simulink model "MX_Gateway"  (Simulink 9.0)
settings: Solver=FixedStepDiscrete  StartTime=0.0  StopTime=100  FixedStep=0.01  RelTol=1e-3
values from neighbouring files (symbols this model uses):
  ACTIVE = Bobcat.Parameter; CreateDefine( ACTIVE                         ,1                          ,'Define' ,'defines.h' )    [defines.m]
  ACTIVE_MODE_ONE = Bobcat.Parameter; CreateDefine( ACTIVE_MODE_ONE                ,19                         ,'Define' ,'defines.h' )    [defines.m]
  AUTHENTICATED = Bobcat.Parameter; CreateDefine( AUTHENTICATED                  ,1                          ,'Define' ,'defines.h' )    [defines.m]
  AUX_EMEA_CONTROLS = Bobcat.Parameter; CreateDefine( AUX_EMEA_CONTROLS  ,1   ,'Define' ,'defines.h' )    [enums.m]
  AUX_FLOW_HIGH = Bobcat.Parameter; CreateDefine( AUX_FLOW_HIGH           ,4  ,'Define' ,'defines.h' )    [enums.m]
  AUX_FLOW_INACTIVE = Bobcat.Parameter; CreateDefine( AUX_FLOW_INACTIVE       ,0  ,'Define' ,'defines.h' )    [enums.m]
  AUX_FLOW_LOW = Bobcat.Parameter; CreateDefine( AUX_FLOW_LOW            ,1  ,'Define' ,'defines.h' )    [enums.m]
  AUX_FLOW_MEDIUM = Bobcat.Parameter; CreateDefine( AUX_FLOW_MEDIUM         ,2  ,'Define' ,'defines.h' )    [enums.m]
  AUX_FLOW_STANDARD = Bobcat.Parameter; CreateDefine( AUX_FLOW_STANDARD       ,3  ,'Define' ,'defines.h' )    [enums.m]
  AUX_NON_FUNCTIONAL = Bobcat.Parameter; CreateDefine( AUX_NON_FUNCTIONAL ,255 ,'Define' ,'defines.h' )    [enums.m]
  BASE_ACTIVE = Bobcat.Parameter; CreateDefine( BASE_ACTIVE                    ,253                        ,'Define' ,'defines.h' )    [defines.m]
  BASE_DETENT = Bobcat.Parameter; CreateDefine( BASE_DETENT                    ,1                          ,'Define' ,'defines.h' )    [defines.m]
  BEEP_NONE = Bobcat.Parameter; CreateDefine( BEEP_NONE                      ,255                        ,'Define' ,'defines.h' )    [defines.m]
  CD_AIR_FILTER_DEBOUNCE_NORMAL = Bobcat.Parameter; CreateAliasCD( CD_AIR_FILTER_DEBOUNCE_NORMAL                        ,uint16(500)                ,'ConstantDataPointer' ,'CONSTANT_DATA' ,'AIR_FILTER_DEBOUNCE_NORMAL')    [cdata.m]    (also in hc12_cdata.m; m4_cdata.m)
  CD_AIR_FILTER_DEBOUNCE_NOT_CONNECTED = Bobcat.Parameter; CreateAliasCD( CD_AIR_FILTER_DEBOUNCE_NOT_CONNECTED                 ,uint16(3000)               ,'ConstantDataPointer' ,'CONSTANT_DATA' ,'AIR_FILTER_DEBOUNCE_NOT_CONNECTED')    [cdata.m]    (also in hc12_cdata.m; m4_cdata.m)
  CD_AIR_FILTER_DEBOUNCE_PLUGGED = Bobcat.Parameter; CreateAliasCD( CD_AIR_FILTER_DEBOUNCE_PLUGGED                       ,uint16(3000)               ,'ConstantDataPointer' ,'CONSTANT_DATA' ,'AIR_FILTER_DEBOUNCE_PLUGGED')    [cdata.m]    (also in hc12_cdata.m; m4_cdata.m)
  CD_AIR_FILTER_FUNCTION = Bobcat.Parameter; CreateAliasCD( CD_AIR_FILTER_FUNCTION                               ,uint8(255)                 ,'ConstantDataPointer' ,'CONSTANT_DATA' ,'AIR_FILTER_FUNCTION')    [cdata.m]    (also in hc12_cdata.m; m4_cdata.m)
  CD_AIR_FILTER_PLUGGED_WEIGHT = Bobcat.Parameter; CreateAliasCD( CD_AIR_FILTER_PLUGGED_WEIGHT                         ,uint8(2)                   ,'ConstantDataPointer' ,'CONSTANT_DATA' ,'AIR_FILTER_PLUGGED_WEIGHT')    [cdata.m]    (also in hc12_cdata.m; m4_cdata.m)
  CD_AIR_FILTER_UNPLUGGED_WEIGHT = Bobcat.Parameter; CreateAliasCD( CD_AIR_FILTER_UNPLUGGED_WEIGHT                       ,uint8(1)                   ,'ConstantDataPointer' ,'CONSTANT_DATA' ,'AIR_FILTER_UNPLUGGED_WEIGHT')    [cdata.m]    (also in hc12_cdata.m; m4_cdata.m)
  CD_AUTOIDLE_ARMBAR_DELAY = Bobcat.Parameter; CreateAliasCD( CD_AUTOIDLE_ARMBAR_DELAY                             ,uint8(2)                   ,'ConstantDataPointer' ,'CONSTANT_DATA' ,'AUTOIDLE_ARMBAR_DELAY')    [cdata.m]    (also in hc12_cdata.m; m4_cdata.m)
  CD_AUTOIDLE_FUNCTION = Bobcat.Parameter; CreateAliasCD( CD_AUTOIDLE_FUNCTION                                 ,uint8(255)                 ,'ConstantDataPointer' ,'CONSTANT_DATA' ,'AUTOIDLE_FUNCTION')    [cdata.m]    (also in hc12_cdata.m; m4_cdata.m)
  CD_AUTOIDLE_IDLE_DELAY = Bobcat.Parameter; CreateAliasCD( CD_AUTOIDLE_IDLE_DELAY                               ,uint8(40)                  ,'ConstantDataPointer' ,'CONSTANT_DATA' ,'AUTOIDLE_IDLE_DELAY')    [cdata.m]    (also in hc12_cdata.m; m4_cdata.m)
  CD_AUTOIDLE_LOW_IDLE_START = Bobcat.Parameter; CreateAliasCD( CD_AUTOIDLE_LOW_IDLE_START                           ,uint8(255)                 ,'ConstantDataPointer' ,'CONSTANT_DATA' ,'AUTOIDLE_LOW_IDLE_START')    [cdata.m]    (also in hc12_cdata.m; m4_cdata.m)
  CD_AUTOIDLE_PRESSURE_OFF = Bobcat.Parameter; CreateAliasCD( CD_AUTOIDLE_PRESSURE_OFF                             ,uint16(300)                ,'ConstantDataPointer' ,'CONSTANT_DATA' ,'AUTOIDLE_PRESSURE_OFF')    [cdata.m]    (also in hc12_cdata.m; m4_cdata.m)
  CD_AUTOIDLE_PRESSURE_ON = Bobcat.Parameter; CreateAliasCD( CD_AUTOIDLE_PRESSURE_ON                              ,uint16(270)                ,'ConstantDataPointer' ,'CONSTANT_DATA' ,'AUTOIDLE_PRESSURE_ON')    [cdata.m]    (also in hc12_cdata.m; m4_cdata.m)
  CD_AUTOSHIFT_FUNCTION = Bobcat.Parameter; CreateAliasCD( CD_AUTOSHIFT_FUNCTION                                ,uint8(255)                 ,'ConstantDataPointer' ,'CONSTANT_DATA' ,'AUTOSHIFT_FUNCTION')    [cdata.m]    (also in hc12_cdata.m; m4_cdata.m)
  CD_AUTOSHIFT_PHIGH1 = Bobcat.Parameter; CreateAliasCD( CD_AUTOSHIFT_PHIGH1                                  ,uint16(205)                ,'ConstantDataPointer' ,'CONSTANT_DATA' ,'AUTOSHIFT_PHIGH1')    [cdata.m]    (also in hc12_cdata.m; m4_cdata.m)
  CD_AUTOSHIFT_PHIGH2 = Bobcat.Parameter; CreateAliasCD( CD_AUTOSHIFT_PHIGH2                                  ,uint16(193)                ,'ConstantDataPointer' ,'CONSTANT_DATA' ,'AUTOSHIFT_PHIGH2')    [cdata.m]    (also in hc12_cdata.m; m4_cdata.m)
  CD_AUTOSHIFT_PHIGH_TEMP1 = Bobcat.Parameter; CreateAliasCD( CD_AUTOSHIFT_PHIGH_TEMP1                             ,int16(70)                  ,'ConstantDataPointer' ,'CONSTANT_DATA' ,'AUTOSHIFT_PHIGH_TEMP1')    [cdata.m]    (also in hc12_cdata.m; m4_cdata.m)
  CD_AUTOSHIFT_PHIGH_TEMP2 = Bobcat.Parameter; CreateAliasCD( CD_AUTOSHIFT_PHIGH_TEMP2                             ,int16(160)                 ,'ConstantDataPointer' ,'CONSTANT_DATA' ,'AUTOSHIFT_PHIGH_TEMP2')    [cdata.m]    (also in hc12_cdata.m; m4_cdata.m)
  CD_AUTOSHIFT_PLOW1 = Bobcat.Parameter; CreateAliasCD( CD_AUTOSHIFT_PLOW1                                   ,uint16(130)                ,'ConstantDataPointer' ,'CONSTANT_DATA' ,'AUTOSHIFT_PLOW1')    [cdata.m]    (also in hc12_cdata.m; m4_cdata.m)
  CD_AUTOSHIFT_PLOW2 = Bobcat.Parameter; CreateAliasCD( CD_AUTOSHIFT_PLOW2                                   ,uint16(100)                ,'ConstantDataPointer' ,'CONSTANT_DATA' ,'AUTOSHIFT_PLOW2')    [cdata.m]    (also in hc12_cdata.m; m4_cdata.m)
  CD_AUTOSHIFT_PLOW_TEMP1 = Bobcat.Parameter; CreateAliasCD( CD_AUTOSHIFT_PLOW_TEMP1                              ,int16(20)                  ,'ConstantDataPointer' ,'CONSTANT_DATA' ,'AUTOSHIFT_PLOW_TEMP1')    [cdata.m]    (also in hc12_cdata.m; m4_cdata.m)
  CD_AUTOSHIFT_PLOW_TEMP2 = Bobcat.Parameter; CreateAliasCD( CD_AUTOSHIFT_PLOW_TEMP2                              ,int16(80)                  ,'ConstantDataPointer' ,'CONSTANT_DATA' ,'AUTOSHIFT_PLOW_TEMP2')    [cdata.m]    (also in hc12_cdata.m; m4_cdata.m)
  CD_AUTOSHIFT_TRAVEL_DELAY = Bobcat.Parameter; CreateAliasCD( CD_AUTOSHIFT_TRAVEL_DELAY                            ,uint8(32)                  ,'ConstantDataPointer' ,'CONSTANT_DATA' ,'AUTOSHIFT_TRAVEL_DELAY')    [cdata.m]    (also in hc12_cdata.m; m4_cdata.m)
  CD_AUX2_CRACKPOINT_CURRENT = Bobcat.Parameter; CreateAliasCD( CD_AUX2_CRACKPOINT_CURRENT                           ,fi(0,0,16,12,'hex','0c28') ,'ConstantDataPointer' ,'CONSTANT_DATA' ,'AUX2_CRACKPOINT_CURRENT')    [cdata.m]    (also in hc12_cdata.m; m4_cdata.m)
  CD_AUX2_FLOW_LOW_FUNCTION = Bobcat.Parameter; CreateAliasCD( CD_AUX2_FLOW_LOW_FUNCTION                            ,uint8(255)                 ,'ConstantDataPointer' ,'CONSTANT_DATA' ,'AUX2_FLOW_LOW_FUNCTION')    [cdata.m]    (also in hc12_cdata.m; m4_cdata.m)
  CD_AUX2_FLOW_MED_FUNCTION = Bobcat.Parameter; CreateAliasCD( CD_AUX2_FLOW_MED_FUNCTION                            ,uint8(255)                 ,'ConstantDataPointer' ,'CONSTANT_DATA' ,'AUX2_FLOW_MED_FUNCTION')    [cdata.m]    (also in hc12_cdata.m; m4_cdata.m)
  CD_AUX2_HI_FLOW_PERCENT = Bobcat.Parameter; CreateAliasCD( CD_AUX2_HI_FLOW_PERCENT                              ,fi(0,0,16,16,'hex','ffff') ,'ConstantDataPointer' ,'CONSTANT_DATA' ,'AUX2_HI_FLOW_PERCENT')    [cdata.m]    (also in hc12_cdata.m; m4_cdata.m)
  CD_AUX2_LOW_FLOW_PERCENT = Bobcat.Parameter; CreateAliasCD( CD_AUX2_LOW_FLOW_PERCENT                             ,fi(0,0,16,16,'hex','7333') ,'ConstantDataPointer' ,'CONSTANT_DATA' ,'AUX2_LOW_FLOW_PERCENT')    [cdata.m]    (also in hc12_cdata.m; m4_cdata.m)
  CD_AUX2_MAX_FLOW_CURRENT = Bobcat.Parameter; CreateAliasCD( CD_AUX2_MAX_FLOW_CURRENT                             ,fi(0,0,16,12,'hex','14cc') ,'ConstantDataPointer' ,'CONSTANT_DATA' ,'AUX2_MAX_FLOW_CURRENT')    [cdata.m]    (also in hc12_cdata.m; m4_cdata.m)
  CD_AUX2_MED_FLOW_PERCENT = Bobcat.Parameter; CreateAliasCD( CD_AUX2_MED_FLOW_PERCENT                             ,fi(0,0,16,16,'hex','9999') ,'ConstantDataPointer' ,'CONSTANT_DATA' ,'AUX2_MED_FLOW_PERCENT')    [cdata.m]    (also in hc12_cdata.m; m4_cdata.m)
  CD_AUX_CRACKPOINT_CURRENT = Bobcat.Parameter; CreateAliasCD( CD_AUX_CRACKPOINT_CURRENT                            ,fi(0,0,16,12,'hex','0000') ,'ConstantDataPointer' ,'CONSTANT_DATA' ,'AUX_CRACKPOINT_CURRENT')    [cdata.m]    (also in hc12_cdata.m; m4_cdata.m)
  CD_AUX_DETENT_FLASH_TIME = Bobcat.Parameter; CreateAliasCD( CD_AUX_DETENT_FLASH_TIME                             ,uint16(72)                 ,'ConstantDataPointer' ,'CONSTANT_DATA' ,'AUX_DETENT_FLASH_TIME')    [cdata.m]    (also in hc12_cdata.m; m4_cdata.m)
  CD_AUX_DETENT_HOLD_TIME = Bobcat.Parameter; CreateAliasCD( CD_AUX_DETENT_HOLD_TIME                              ,uint16(100)                ,'ConstantDataPointer' ,'CONSTANT_DATA' ,'AUX_DETENT_HOLD_TIME')    [cdata.m]    (also in hc12_cdata.m; m4_cdata.m)
  CD_AUX_FULL_ON_AUX1 = Bobcat.Parameter; CreateAliasCD( CD_AUX_FULL_ON_AUX1                                  ,fi(0,0,16,12,'hex','10cd') ,'ConstantDataPointer' ,'CONSTANT_DATA' ,'AUX_FULL_ON_AUX1')    [cdata.m]    (also in hc12_cdata.m; m4_cdata.m)
  CD_AUX_FULL_ON_AUX2 = Bobcat.Parameter; CreateAliasCD( CD_AUX_FULL_ON_AUX2                                  ,fi(0,0,16,12,'hex','139d') ,'ConstantDataPointer' ,'CONSTANT_DATA' ,'AUX_FULL_ON_AUX2')    [cdata.m]    (also in hc12_cdata.m; m4_cdata.m)
  CD_AUX_FULL_ON_AUX3 = Bobcat.Parameter; CreateAliasCD( CD_AUX_FULL_ON_AUX3                                  ,fi(0,0,16,12,'hex','17d7') ,'ConstantDataPointer' ,'CONSTANT_DATA' ,'AUX_FULL_ON_AUX3')    [cdata.m]    (also in hc12_cdata.m; m4_cdata.m)
  CD_AUX_FUNCTION = Bobcat.Parameter; CreateAliasCD( CD_AUX_FUNCTION                                      ,uint8(0)                   ,'ConstantDataPointer' ,'CONSTANT_DATA' ,'AUX_FUNCTION')    [cdata.m]    (also in hc12_cdata.m; m4_cdata.m)
  CD_AUX_RATE_LIMIT_CRACKPOINT = Bobcat.Parameter; CreateAliasCD( CD_AUX_RATE_LIMIT_CRACKPOINT                         ,fi(0,0,16,12,'hex','0800') ,'ConstantDataPointer' ,'CONSTANT_DATA' ,'AUX_RATE_LIMIT_CRACKPOINT')    [cdata.m]    (also in hc12_cdata.m; m4_cdata.m)
  CD_AUX_RATE_LIMIT_CRACKPOINT_RATE = Bobcat.Parameter; CreateAliasCD( CD_AUX_RATE_LIMIT_CRACKPOINT_RATE                    ,fi(0,1,32,12,'int',102400) ,'ConstantDataPointer' ,'CONSTANT_DATA' ,'AUX_RATE_LIMIT_CRACKPOINT_RATE')    [cdata.m]    (also in hc12_cdata.m; m4_cdata.m)
  CD_AUX_RATE_LIMIT_FUNCTION = Bobcat.Parameter; CreateAliasCD( CD_AUX_RATE_LIMIT_FUNCTION                           ,uint8(255)                 ,'ConstantDataPointer' ,'CONSTANT_DATA' ,'AUX_RATE_LIMIT_FUNCTION')    [cdata.m]    (also in hc12_cdata.m; m4_cdata.m)
  CD_AUX_RATE_LIMIT_MAX_ENG_SPD = Bobcat.Parameter; CreateAliasCD( CD_AUX_RATE_LIMIT_MAX_ENG_SPD                        ,uint16(1600)               ,'ConstantDataPointer' ,'CONSTANT_DATA' ,'AUX_RATE_LIMIT_MAX_ENG_SPD')    [cdata.m]    (also in hc12_cdata.m; m4_cdata.m)
  CD_AUX_RATE_LIMIT_MIN_ENG_SPD = Bobcat.Parameter; CreateAliasCD( CD_AUX_RATE_LIMIT_MIN_ENG_SPD                        ,uint16(100)                ,'ConstantDataPointer' ,'CONSTANT_DATA' ,'AUX_RATE_LIMIT_MIN_ENG_SPD')    [cdata.m]    (also in hc12_cdata.m; m4_cdata.m)
  CD_AUX_RATE_LIMIT_NORMAL_RATE = Bobcat.Parameter; CreateAliasCD( CD_AUX_RATE_LIMIT_NORMAL_RATE                        ,fi(0,1,32,12,'int',2048)   ,'ConstantDataPointer' ,'CONSTANT_DATA' ,'AUX_RATE_LIMIT_NORMAL_RATE')    [cdata.m]    (also in hc12_cdata.m; m4_cdata.m)
  CD_AUXPOT_CAL_DEADBAND_HIGH = Bobcat.Parameter; CreateAliasCD( CD_AUXPOT_CAL_DEADBAND_HIGH                          ,uint16(574)                ,'ConstantDataPointer' ,'CONSTANT_DATA' ,'AUXPOT_CAL_DEADBAND_HIGH')    [cdata.m]    (also in hc12_cdata.m; m4_cdata.m)
  CD_AUXPOT_CAL_DEADBAND_LOW = Bobcat.Parameter; CreateAliasCD( CD_AUXPOT_CAL_DEADBAND_LOW                           ,uint16(424)                ,'ConstantDataPointer' ,'CONSTANT_DATA' ,'AUXPOT_CAL_DEADBAND_LOW')    [cdata.m]    (also in hc12_cdata.m; m4_cdata.m)
  CD_AUXPOT_CENTER_RATIO = Bobcat.Parameter; CreateAliasCD( CD_AUXPOT_CENTER_RATIO                               ,uint16(500)                ,'ConstantDataPointer' ,'CONSTANT_DATA' ,'AUXPOT_CENTER_RATIO')    [cdata.m]    (also in hc12_cdata.m; m4_cdata.m)
  CD_AUXPOT_DEADBAND = Bobcat.Parameter; CreateAliasCD( CD_AUXPOT_DEADBAND                                   ,uint8(38)                  ,'ConstantDataPointer' ,'CONSTANT_DATA' ,'AUXPOT_DEADBAND')    [cdata.m]    (also in hc12_cdata.m; m4_cdata.m)
  CD_AUXPOT_FUNCTION = Bobcat.Parameter; CreateAliasCD( CD_AUXPOT_FUNCTION                                   ,uint8(0)                   ,'ConstantDataPointer' ,'CONSTANT_DATA' ,'AUXPOT_FUNCTION')    [cdata.m]    (also in hc12_cdata.m; m4_cdata.m)
  CD_AUXPOT_RATIO_GAIN = Bobcat.Parameter; CreateAliasCD( CD_AUXPOT_RATIO_GAIN                                 ,uint16(1000)               ,'ConstantDataPointer' ,'CONSTANT_DATA' ,'AUXPOT_RATIO_GAIN')    [cdata.m]    (also in hc12_cdata.m; m4_cdata.m)
  CD_CAN_THROTTLE_FUNCTION = Bobcat.Parameter; CreateAliasCD( CD_CAN_THROTTLE_FUNCTION                             ,uint8(255)                 ,'ConstantDataPointer' ,'CONSTANT_DATA' ,'CAN_THROTTLE_FUNCTION')    [cdata.m]    (also in hc12_cdata.m; m4_cdata.m)
  CD_CAN_THROTTLE_REVERSE = Bobcat.Parameter; CreateAliasCD( CD_CAN_THROTTLE_REVERSE                              ,uint8(255)                 ,'ConstantDataPointer' ,'CONSTANT_DATA' ,'CAN_THROTTLE_REVERSE')    [cdata.m]    (also in hc12_cdata.m; m4_cdata.m)
  CD_CONSOLE_DEBOUNCE_ABSENT = Bobcat.Parameter; CreateAliasCD( CD_CONSOLE_DEBOUNCE_ABSENT                           ,uint16(12)                 ,'ConstantDataPointer' ,'CONSTANT_DATA' ,'CONSOLE_DEBOUNCE_ABSENT')    [cdata.m]    (also in hc12_cdata.m; m4_cdata.m)
  CD_CONSOLE_DEBOUNCE_OORH = Bobcat.Parameter; CreateAliasCD( CD_CONSOLE_DEBOUNCE_OORH                             ,uint16(12)                 ,'ConstantDataPointer' ,'CONSTANT_DATA' ,'CONSOLE_DEBOUNCE_OORH')    [cdata.m]    (also in hc12_cdata.m; m4_cdata.m)
  CD_CONSOLE_DEBOUNCE_OORL = Bobcat.Parameter; CreateAliasCD( CD_CONSOLE_DEBOUNCE_OORL                             ,uint16(12)                 ,'ConstantDataPointer' ,'CONSTANT_DATA' ,'CONSOLE_DEBOUNCE_OORL')    [cdata.m]    (also in hc12_cdata.m; m4_cdata.m)
  CD_CONSOLE_DEBOUNCE_PRESENT = Bobcat.Parameter; CreateAliasCD( CD_CONSOLE_DEBOUNCE_PRESENT                          ,uint16(12)                 ,'ConstantDataPointer' ,'CONSTANT_DATA' ,'CONSOLE_DEBOUNCE_PRESENT')    [cdata.m]    (also in hc12_cdata.m; m4_cdata.m)
  CD_CONSOLE_FUNCTION = Bobcat.Parameter; CreateAliasCD( CD_CONSOLE_FUNCTION                                  ,uint8(0)                   ,'ConstantDataPointer' ,'CONSTANT_DATA' ,'CONSOLE_FUNCTION')    [cdata.m]    (also in hc12_cdata.m; m4_cdata.m)
  CD_CONSOLE_OORH = Bobcat.Parameter; CreateAliasCD( CD_CONSOLE_OORH                                      ,uint16(740)                ,'ConstantDataPointer' ,'CONSTANT_DATA' ,'CONSOLE_OORH')    [cdata.m]    (also in hc12_cdata.m; m4_cdata.m)
  CD_CONSOLE_OORL = Bobcat.Parameter; CreateAliasCD( CD_CONSOLE_OORL                                      ,uint16(10)                 ,'ConstantDataPointer' ,'CONSTANT_DATA' ,'CONSOLE_OORL')    [cdata.m]    (also in hc12_cdata.m; m4_cdata.m)
  CD_CONSOLE_PRESENT_HIGH = Bobcat.Parameter; CreateAliasCD( CD_CONSOLE_PRESENT_HIGH                              ,uint16(515)                ,'ConstantDataPointer' ,'CONSTANT_DATA' ,'CONSOLE_PRESENT_HIGH')    [cdata.m]    (also in hc12_cdata.m; m4_cdata.m)
  CD_CONSOLE_PRESENT_LOW = Bobcat.Parameter; CreateAliasCD( CD_CONSOLE_PRESENT_LOW                               ,uint16(375)                ,'ConstantDataPointer' ,'CONSTANT_DATA' ,'CONSOLE_PRESENT_LOW')    [cdata.m]    (also in hc12_cdata.m; m4_cdata.m)
  CD_DELUXE_GEN5_5_FUNCTION = Bobcat.Parameter; CreateAliasCD( CD_DELUXE_GEN5_5_FUNCTION                            ,uint8(255)                 ,'ConstantDataPointer' ,'CONSTANT_DATA' ,'DELUXE_GEN5_5_FUNCTION')    [cdata.m]    (also in hc12_cdata.m; m4_cdata.m)
  CD_DELUXE_GEN5_FUNCTION = Bobcat.Parameter; CreateAliasCD( CD_DELUXE_GEN5_FUNCTION                              ,uint8(255)                 ,'ConstantDataPointer' ,'CONSTANT_DATA' ,'DELUXE_GEN5_FUNCTION')    [cdata.m]    (also in hc12_cdata.m; m4_cdata.m)
  CD_DERATE_ENGINE_SPEED_DEBOUNCE_HIGH = Bobcat.Parameter; CreateAliasCD( CD_DERATE_ENGINE_SPEED_DEBOUNCE_HIGH                 ,uint16(200)                ,'ConstantDataPointer' ,'CONSTANT_DATA' ,'DERATE_ENGINE_SPEED_DEBOUNCE_HIGH')    [cdata.m]    (also in hc12_cdata.m; m4_cdata.m)
  CD_DERATE_ENGINE_SPEED_DEBOUNCE_SHUTDOWN = Bobcat.Parameter; CreateAliasCD( CD_DERATE_ENGINE_SPEED_DEBOUNCE_SHUTDOWN             ,uint16(1000)               ,'ConstantDataPointer' ,'CONSTANT_DATA' ,'DERATE_ENGINE_SPEED_DEBOUNCE_SHUTDOWN')    [cdata.m]    (also in hc12_cdata.m; m4_cdata.m)
  CD_DERATE_ENGINE_SPEED_HIGH_OFFSET = Bobcat.Parameter; CreateAliasCD( CD_DERATE_ENGINE_SPEED_HIGH_OFFSET                   ,uint16(200)                ,'ConstantDataPointer' ,'CONSTANT_DATA' ,'DERATE_ENGINE_SPEED_HIGH_OFFSET')    [cdata.m]    (also in hc12_cdata.m; m4_cdata.m)
  CD_DERATE_METHOD = Bobcat.Parameter; CreateAliasCD( CD_DERATE_METHOD                                     ,uint8(0)                   ,'ConstantDataPointer' ,'CONSTANT_DATA' ,'DERATE_METHOD')    [cdata.m]    (also in hc12_cdata.m; m4_cdata.m)
  CD_DUMP_VALVE_FUNCTION = Bobcat.Parameter; CreateAliasCD( CD_DUMP_VALVE_FUNCTION                               ,uint8(255)                 ,'ConstantDataPointer' ,'CONSTANT_DATA' ,'DUMP_VALVE_FUNCTION')    [cdata.m]    (also in hc12_cdata.m; m4_cdata.m)
  CD_DUMP_VALVE_ON_CURRENT = Bobcat.Parameter; CreateAliasCD( CD_DUMP_VALVE_ON_CURRENT                             ,fi(0,0,16,12,'hex','ffff') ,'ConstantDataPointer' ,'CONSTANT_DATA' ,'DUMP_VALVE_ON_CURRENT')    [cdata.m]    (also in hc12_cdata.m; m4_cdata.m)
  CD_ECO_MODE_THROTTLE_REDUCTION = Bobcat.Parameter; CreateAliasCD( CD_ECO_MODE_THROTTLE_REDUCTION                       ,uint16(850)                ,'ConstantDataPointer' ,'CONSTANT_DATA' ,'ECO_MODE_THROTTLE_REDUCTION')    [cdata.m]    (also in hc12_cdata.m; m4_cdata.m)
  CD_ECT_FILTER_GAIN = Bobcat.Parameter; CreateAliasCD( CD_ECT_FILTER_GAIN                                   ,uint8(6)                   ,'ConstantDataPointer' ,'CONSTANT_DATA' ,'ECT_FILTER_GAIN')    [cdata.m]    (also in hc12_cdata.m; m4_cdata.m)
  CD_ECU_FUNCTION = Bobcat.Parameter; CreateAliasCD( CD_ECU_FUNCTION                                      ,uint8(255)                 ,'ConstantDataPointer' ,'CONSTANT_DATA' ,'ECU_FUNCTION')    [cdata.m]    (also in hc12_cdata.m; m4_cdata.m)
  CD_ECU_HOLD_DEBOUNCE_ERROR_ON = Bobcat.Parameter; CreateAliasCD( CD_ECU_HOLD_DEBOUNCE_ERROR_ON                        ,uint16(20)                 ,'ConstantDataPointer' ,'CONSTANT_DATA' ,'ECU_HOLD_DEBOUNCE_ERROR_ON')    [cdata.m]    (also in hc12_cdata.m; m4_cdata.m)
  CD_EMEA_CONTROLS_HOLD_TIME = Bobcat.Parameter; CreateAliasCD( CD_EMEA_CONTROLS_HOLD_TIME                           ,uint16(30)                 ,'ConstantDataPointer' ,'CONSTANT_DATA' ,'EMEA_CONTROLS_HOLD_TIME')    [cdata.m]    (also in hc12_cdata.m; m4_cdata.m)
  CD_EMEA_CONTROLS_OPTION_A = Bobcat.Parameter; CreateAliasCD( CD_EMEA_CONTROLS_OPTION_A                            ,uint8(255)                 ,'ConstantDataPointer' ,'CONSTANT_DATA' ,'EMEA_CONTROLS_OPTION_A')    [cdata.m]    (also in hc12_cdata.m; m4_cdata.m)
  CD_EMEA_CONTROLS_OPTION_C = Bobcat.Parameter; CreateAliasCD( CD_EMEA_CONTROLS_OPTION_C                            ,uint8(255)                 ,'ConstantDataPointer' ,'CONSTANT_DATA' ,'EMEA_CONTROLS_OPTION_C')    [cdata.m]    (also in hc12_cdata.m; m4_cdata.m)
  CD_EMEA_CONTROLS_REVERSE = Bobcat.Parameter; CreateAliasCD( CD_EMEA_CONTROLS_REVERSE                             ,uint8(255)                 ,'ConstantDataPointer' ,'CONSTANT_DATA' ,'EMEA_CONTROLS_REVERSE')    [cdata.m]    (also in hc12_cdata.m; m4_cdata.m)
  CD_EMEA_ELECTRIC_VERSION = Bobcat.Parameter; CreateAliasCD( CD_EMEA_ELECTRIC_VERSION                             ,uint8(0)                   ,'ConstantDataPointer' ,'CONSTANT_DATA' ,'EMEA_ELECTRIC_VERSION')    [cdata.m]    (also in hc12_cdata.m; m4_cdata.m)
  CD_EMEA_POT_CAL_DEADBAND_HIGH = Bobcat.Parameter; CreateAliasCD( CD_EMEA_POT_CAL_DEADBAND_HIGH                        ,uint16(574)                ,'ConstantDataPointer' ,'CONSTANT_DATA' ,'EMEA_POT_CAL_DEADBAND_HIGH')    [cdata.m]    (also in hc12_cdata.m; m4_cdata.m)
  CD_EMEA_POT_CAL_DEADBAND_LOW = Bobcat.Parameter; CreateAliasCD( CD_EMEA_POT_CAL_DEADBAND_LOW                         ,uint16(424)                ,'ConstantDataPointer' ,'CONSTANT_DATA' ,'EMEA_POT_CAL_DEADBAND_LOW')    [cdata.m]    (also in hc12_cdata.m; m4_cdata.m)
  CD_ENGINE_RPM_FACTOR = Bobcat.Parameter; CreateAliasCD( CD_ENGINE_RPM_FACTOR                                 ,uint8(35)                  ,'ConstantDataPointer' ,'CONSTANT_DATA' ,'ENGINE_RPM_FACTOR')    [cdata.m]    (also in hc12_cdata.m; m4_cdata.m)
  CD_ENGINE_SPEED_FILTER_FAST_VALUE = Bobcat.Parameter; CreateAliasCD( CD_ENGINE_SPEED_FILTER_FAST_VALUE                    ,uint8(1)                   ,'ConstantDataPointer' ,'CONSTANT_DATA' ,'ENGINE_SPEED_FILTER_FAST_VALUE')    [cdata.m]    (also in hc12_cdata.m; m4_cdata.m)
  CD_ENGINE_SPEED_FILTER_SLOW_VALUE = Bobcat.Parameter; CreateAliasCD( CD_ENGINE_SPEED_FILTER_SLOW_VALUE                    ,uint8(4)                   ,'ConstantDataPointer' ,'CONSTANT_DATA' ,'ENGINE_SPEED_FILTER_SLOW_VALUE')    [cdata.m]    (also in hc12_cdata.m; m4_cdata.m)
  CD_ENGINE_SPEED_RUNNING = Bobcat.Parameter; CreateAliasCD( CD_ENGINE_SPEED_RUNNING                              ,uint16(800)                ,'ConstantDataPointer' ,'CONSTANT_DATA' ,'ENGINE_SPEED_RUNNING')    [cdata.m]    (also in hc12_cdata.m; m4_cdata.m)
  CD_ENGINE_START_DELAY_DEBOUNCE_RPM_NO_SIGNAL = Bobcat.Parameter; CreateAliasCD( CD_ENGINE_START_DELAY_DEBOUNCE_RPM_NO_SIGNAL         ,uint16(0)                  ,'ConstantDataPointer' ,'CONSTANT_DATA' ,'ENGINE_START_DELAY_DEBOUNCE_RPM_NO_SIGNAL')    [cdata.m]    (also in hc12_cdata.m; m4_cdata.m)
  CD_ENGINE_START_DELAY_OFF_TIME = Bobcat.Parameter; CreateAliasCD( CD_ENGINE_START_DELAY_OFF_TIME                       ,uint16(0)                  ,'ConstantDataPointer' ,'CONSTANT_DATA' ,'ENGINE_START_DELAY_OFF_TIME')    [cdata.m]    (also in hc12_cdata.m; m4_cdata.m)
  CD_ENGINE_START_DELAY_RPM_NO_SIGNAL = Bobcat.Parameter; CreateAliasCD( CD_ENGINE_START_DELAY_RPM_NO_SIGNAL                  ,uint16(0)                  ,'ConstantDataPointer' ,'CONSTANT_DATA' ,'ENGINE_START_DELAY_RPM_NO_SIGNAL')    [cdata.m]    (also in hc12_cdata.m; m4_cdata.m)
  CD_EOP_DEBOUNCE_EXTREMELY_LOW = Bobcat.Parameter; CreateAliasCD( CD_EOP_DEBOUNCE_EXTREMELY_LOW                        ,uint16(50)                 ,'ConstantDataPointer' ,'CONSTANT_DATA' ,'EOP_DEBOUNCE_EXTREMELY_LOW')    [cdata.m]    (also in hc12_cdata.m; m4_cdata.m)
  CD_EOP_DEBOUNCE_NORMAL = Bobcat.Parameter; CreateAliasCD( CD_EOP_DEBOUNCE_NORMAL                               ,uint16(50)                 ,'ConstantDataPointer' ,'CONSTANT_DATA' ,'EOP_DEBOUNCE_NORMAL')    [cdata.m]    (also in hc12_cdata.m; m4_cdata.m)
  CD_EOP_DEBOUNCE_SHUTDOWN = Bobcat.Parameter; CreateAliasCD( CD_EOP_DEBOUNCE_SHUTDOWN                             ,uint16(100)                ,'ConstantDataPointer' ,'CONSTANT_DATA' ,'EOP_DEBOUNCE_SHUTDOWN')    [cdata.m]    (also in hc12_cdata.m; m4_cdata.m)
  CD_EOP_FILTER_VALUE = Bobcat.Parameter; CreateAliasCD( CD_EOP_FILTER_VALUE                                  ,uint8(3)                   ,'ConstantDataPointer' ,'CONSTANT_DATA' ,'EOP_FILTER_VALUE')    [cdata.m]    (also in hc12_cdata.m; m4_cdata.m)
  CD_EOP_FUNCTION = Bobcat.Parameter; CreateAliasCD( CD_EOP_FUNCTION                                      ,uint8(1)                   ,'ConstantDataPointer' ,'CONSTANT_DATA' ,'EOP_FUNCTION')    [cdata.m]    (also in hc12_cdata.m; m4_cdata.m)
  CD_EOP_SWITCH_GAUGE_VALUE = Bobcat.Parameter; CreateAliasCD( CD_EOP_SWITCH_GAUGE_VALUE                            ,uint16(125)                ,'ConstantDataPointer' ,'CONSTANT_DATA' ,'EOP_SWITCH_GAUGE_VALUE')    [cdata.m]    (also in hc12_cdata.m; m4_cdata.m)
  CD_EOP_SWITCH_LOW = Bobcat.Parameter; CreateAliasCD( CD_EOP_SWITCH_LOW                                    ,uint16(50)                 ,'ConstantDataPointer' ,'CONSTANT_DATA' ,'EOP_SWITCH_LOW')    [cdata.m]    (also in hc12_cdata.m; m4_cdata.m)
  CD_EOP_SWITCH_PRESSURE_VALUE = Bobcat.Parameter; CreateAliasCD( CD_EOP_SWITCH_PRESSURE_VALUE                         ,uint16(40)                 ,'ConstantDataPointer' ,'CONSTANT_DATA' ,'EOP_SWITCH_PRESSURE_VALUE')    [cdata.m]    (also in hc12_cdata.m; m4_cdata.m)
  CD_EOT_DEBOUNCE_EXTREMELY_LOW = Bobcat.Parameter; CreateAliasCD( CD_EOT_DEBOUNCE_EXTREMELY_LOW                        ,uint16(2)                  ,'ConstantDataPointer' ,'CONSTANT_DATA' ,'EOT_DEBOUNCE_EXTREMELY_LOW')    [cdata.m]    (also in hc12_cdata.m; m4_cdata.m)
  CD_EOT_DEBOUNCE_LOW = Bobcat.Parameter; CreateAliasCD( CD_EOT_DEBOUNCE_LOW                                  ,uint16(2)                  ,'ConstantDataPointer' ,'CONSTANT_DATA' ,'EOT_DEBOUNCE_LOW')    [cdata.m]    (also in hc12_cdata.m; m4_cdata.m)
  CD_EOT_DEBOUNCE_NORMAL = Bobcat.Parameter; CreateAliasCD( CD_EOT_DEBOUNCE_NORMAL                               ,uint16(2)                  ,'ConstantDataPointer' ,'CONSTANT_DATA' ,'EOT_DEBOUNCE_NORMAL')    [cdata.m]    (also in hc12_cdata.m; m4_cdata.m)
  CD_EOT_EXTREMELY_LOW = Bobcat.Parameter; CreateAliasCD( CD_EOT_EXTREMELY_LOW                                 ,uint16(81)                 ,'ConstantDataPointer' ,'CONSTANT_DATA' ,'EOT_EXTREMELY_LOW')    [cdata.m]    (also in hc12_cdata.m; m4_cdata.m)
  CD_EOT_FUNCTION = Bobcat.Parameter; CreateAliasCD( CD_EOT_FUNCTION                                      ,uint8(255)                 ,'ConstantDataPointer' ,'CONSTANT_DATA' ,'EOT_FUNCTION')    [cdata.m]    (also in hc12_cdata.m; m4_cdata.m)
  CD_EOT_LOW = Bobcat.Parameter; CreateAliasCD( CD_EOT_LOW                                           ,uint16(90)                 ,'ConstantDataPointer' ,'CONSTANT_DATA' ,'EOT_LOW')    [cdata.m]    (also in hc12_cdata.m; m4_cdata.m)
  CD_EOT_TEMPERATURE_HYSTERESIS = Bobcat.Parameter; CreateAliasCD( CD_EOT_TEMPERATURE_HYSTERESIS                        ,uint16(2)                  ,'ConstantDataPointer' ,'CONSTANT_DATA' ,'EOT_TEMPERATURE_HYSTERESIS')    [cdata.m]    (also in hc12_cdata.m; m4_cdata.m)
  CD_FAST_TRAVEL_FUNCTION = Bobcat.Parameter; CreateAliasCD( CD_FAST_TRAVEL_FUNCTION                              ,uint8(255)                 ,'ConstantDataPointer' ,'CONSTANT_DATA' ,'FAST_TRAVEL_FUNCTION')    [cdata.m]    (also in hc12_cdata.m; m4_cdata.m)
  CD_FAST_TRAVEL_THROTTLE_DELAY = Bobcat.Parameter; CreateAliasCD( CD_FAST_TRAVEL_THROTTLE_DELAY                        ,uint16(10)                 ,'ConstantDataPointer' ,'CONSTANT_DATA' ,'FAST_TRAVEL_THROTTLE_DELAY')    [cdata.m]    (also in hc12_cdata.m; m4_cdata.m)
  CD_FAST_TRAVEL_THROTTLE_LIMIT = Bobcat.Parameter; CreateAliasCD( CD_FAST_TRAVEL_THROTTLE_LIMIT                        ,uint16(1000)               ,'ConstantDataPointer' ,'CONSTANT_DATA' ,'FAST_TRAVEL_THROTTLE_LIMIT')    [cdata.m]    (also in hc12_cdata.m; m4_cdata.m)
  CD_FLYWHEEL_TEETH = Bobcat.Parameter; CreateAliasCD( CD_FLYWHEEL_TEETH                                    ,uint8(83)                  ,'ConstantDataPointer' ,'CONSTANT_DATA' ,'FLYWHEEL_TEETH')    [cdata.m]    (also in hc12_cdata.m; m4_cdata.m)
  CD_FUEL_LEVEL_FILTER_FAST_VALUE = Bobcat.Parameter; CreateAliasCD( CD_FUEL_LEVEL_FILTER_FAST_VALUE                      ,uint8(5)                   ,'ConstantDataPointer' ,'CONSTANT_DATA' ,'FUEL_LEVEL_FILTER_FAST_VALUE')    [cdata.m]    (also in hc12_cdata.m; m4_cdata.m)
  CD_FUEL_LEVEL_FILTER_SLOW_VALUE = Bobcat.Parameter; CreateAliasCD( CD_FUEL_LEVEL_FILTER_SLOW_VALUE                      ,uint8(10)                  ,'ConstantDataPointer' ,'CONSTANT_DATA' ,'FUEL_LEVEL_FILTER_SLOW_VALUE')    [cdata.m]    (also in hc12_cdata.m; m4_cdata.m)
  CD_FUEL_PRIMARY_FEEDBACK_FUNCTION = Bobcat.Parameter; CreateAliasCD( CD_FUEL_PRIMARY_FEEDBACK_FUNCTION                    ,uint8(0)                   ,'ConstantDataPointer' ,'CONSTANT_DATA' ,'FUEL_PRIMARY_FEEDBACK_FUNCTION')    [cdata.m]    (also in hc12_cdata.m; m4_cdata.m)
  ... (+246 more)
undefined symbols (not defined in the file or in its folder): sigbuilder_block
undefined symbols (not defined in the file): CD_FUEL_RESOLUTION_TIMER
libraries: simulink x186, MX_Library x107
(part 16 of 48)

== system MX_Gateway/MX_Gtwy_Control/Outputs/Lights ==
  dfbk_LightsOutput : Inport  OutDataTypeStr=boolean
  din_LightsRelay : Inport  Port=2  OutDataTypeStr=boolean
  button_lights : Inport  Port=3
  state_Buttons : Inport  Port=4  OutDataTypeStr=uint8
  state_VBattery : Inport  Port=5  OutDataTypeStr=uint8
  Compare To Constant1 : simulink/Logic and Bit Operations/Compare To Constant  relop=~=  const=NON_FUNCTIONAL  OutDataTypeStr=boolean  ZeroCross=off
  Compare To Constant2 : simulink/Logic and Bit Operations/Compare To Constant  relop=~=  const=OORL  OutDataTypeStr=boolean  ZeroCross=off
  Compare To Constant3 : simulink/Logic and Bit Operations/Compare To Constant  relop=~=  const=NON_FUNCTIONAL  OutDataTypeStr=boolean  ZeroCross=off
  Compare To Constant4 : simulink/Logic and Bit Operations/Compare To Constant  relop=~=  const=OORL  OutDataTypeStr=boolean  ZeroCross=off
  Light1FeedbackState : Constant  Value=CD_LIGHTS_FEEDBACK_FUNCTION  OutDataTypeStr=uint8
  Light1FeedbackState1 : Constant  Value=CD_LIGHTS_RELAY_FEEDBACK_FUNCTION  OutDataTypeStr=uint8
  Lights : Stateflow chart  TreatAsAtomicUnit=on
  Logical Operator1 : Logic  AllPortsSameDT=off  OutDataTypeStr=boolean
  Logical Operator2 : Logic  AllPortsSameDT=off  OutDataTypeStr=boolean
  Logical Operator3 : Logic  Operator=NOT  AllPortsSameDT=off  OutDataTypeStr=boolean
  Switch : Switch  Criteria=u2 ~= 0
  Unit Delay1 : UnitDelay  InitialCondition=NORMAL  SampleTime=-1
      settings: AttributesFormatString=<initial=%<X0>>\n<tsample=%<SampleTime>>
  Unit Delay3 : UnitDelay  InitialCondition=NORMAL  SampleTime=-1
      settings: AttributesFormatString=<initial=%<X0>>\n<tsample=%<SampleTime>>
  dout_chk : MX_Library/dout_chk  ERR_ON_DB_CNT=CD_LIGHTS_DEBOUNCE_ERROR_ON  ERR_OFF_DB_CNT=CD_LIGHTS_DEBOUNCE_ERROR_OFF
  relay_oc : MX_Library/relay_oc  ERROR_ON_DB_CNT=CD_LIGHTS_DEBOUNCE_ERROR_ON  ERROR_OFF_DB_CNT=CD_LIGHTS_DEBOUNCE_ERROR_OFF  NORMAL_DB_CNT=CD_LIGHTS_DEBOUNCE_NORMAL
  dout_Lights : Outport
  state_LightsOutput : Outport  Port=2
  state_LightsRelay : Outport  Port=3
  wires:
  Lights/1(dout_Lights) -> dout_chk/1, dout_Lights/1, relay_oc/1
  [state_VBattery] -> Lights/1(state_VBattery)  "state_VBattery"
  state_VBattery/1 -> [state_VBattery]  "state_VBattery"
  Logical Operator2/1 -> dout_chk/Enable  "ErrorDetectionEnable"
  Light1FeedbackState/1 -> Compare To Constant1/1
  [state_VBattery] -> Compare To Constant2/1  "state_VBattery"
  Compare To Constant1/1 -> Logical Operator2/1
  Compare To Constant2/1 -> Logical Operator2/2
  state_Buttons/1 -> Lights/2(state_Buttons)
  button_lights/1 -> Lights/3(button_Lights)
  din_LightsRelay/1 -> relay_oc/3
  Light1FeedbackState1/1 -> Compare To Constant3/1
  [state_VBattery] -> Compare To Constant4/1  "state_VBattery"
  Compare To Constant3/1 -> Logical Operator1/1
  Compare To Constant4/1 -> Logical Operator1/2
  Logical Operator1/1 -> relay_oc/Enable, Logical Operator3/1
  relay_oc/2 -> state_LightsRelay/1, [state_LightsRelay]
  Switch/1 -> state_LightsOutput/1, [state_LightsOutput]
  dout_chk/1 -> Switch/1
  relay_oc/1 -> Switch/3
  Logical Operator3/1 -> Switch/2
  dfbk_LightsOutput/1 -> [dfbk_LightsOutput]
  [dfbk_LightsOutput] -> relay_oc/2
  [dfbk_LightsOutput] -> dout_chk/2
  Unit Delay3/1 -> Lights/4(state_LightsOutput)
  [state_LightsOutput] -> Unit Delay3/1
  [state_LightsRelay] -> Unit Delay1/1
  Unit Delay1/1 -> Lights/5(state_LightsRelay)

== system MX_Gateway/MX_Gtwy_Control/Outputs/Offset ==
  state_Engine : Inport
  mode_LeftControl : Inport  Port=2
  mode_RightControl : Inport  Port=3
  state_Console : Inport  Port=4
  state_Vbattery : Inport  Port=5
  calc_Vbattery : Inport  Port=6
  position_OffsetPot : Inport  Port=7
  state_OffsetPot : Inport  Port=8
  position_LeftPot : Inport  Port=9
  state_LeftPot : Inport  Port=10
  position_RightPot : Inport  Port=11
  state_RightPot : Inport  Port=12
  state_System : Inport  Port=13
  afbk_A2D_OffsetBase : Inport  Port=14
  ifbk_A2D_OffsetBase : Inport  Port=15
  afbk_A2D_OffsetRod : Inport  Port=16
  ifbk_A2D_OffsetRod : Inport  Port=17
  ain_PrecRef : Inport  Port=18
  flow_Aux2Percent : Inport  Port=19
  flow_OffsetPercent : Inport  Port=20
  state_Diverter : Inport  Port=21
  CompareToConstant : simulink/Logic and Bit Operations/Compare To Constant  relop=~=  const=NON_FUNCTIONAL  OutDataTypeStr=boolean  ZeroCross=off
  CompareToConstant1 : simulink/Logic and Bit Operations/Compare To Constant  relop===  const=SYS_NORMAL  OutDataTypeStr=boolean  ZeroCross=off
  Constant : Constant  Value=CD_OFFSET_FUNCTION
  Logical Operator : Logic  AllPortsSameDT=off  OutDataTypeStr=boolean
  Offset : Subsystem
  hs_OffsetBase : Outport  OutputWhenDisabled=reset  InitialOutput=[0]
  ls_OffsetBase : Outport  Port=2  OutputWhenDisabled=reset  InitialOutput=[0]
  errorCC_OffsetBase : Outport  Port=3  OutputWhenDisabled=reset  InitialOutput=[0]
  calc_ifbk_OffsetBase : Outport  Port=4  OutputWhenDisabled=reset  InitialOutput=[0]
  state_OffsetBase : Outport  Port=5  OutputWhenDisabled=reset  InitialOutput=[0]
  hs_OffsetRod : Outport  Port=6  OutputWhenDisabled=reset  InitialOutput=[0]
  ls_OffsetRod : Outport  Port=7  OutputWhenDisabled=reset  InitialOutput=[INIT_NORMAL]
  errorCC_OffsetRod : Outport  Port=8  OutputWhenDisabled=reset  InitialOutput=[0]
  calc_ifbk_OffsetRod : Outport  Port=9  OutputWhenDisabled=reset  InitialOutput=[INIT_NORMAL]
  state_OffsetRod : Outport  Port=10
  wires:
  state_System/1 -> CompareToConstant1/1
  CompareToConstant1/1 -> Logical Operator/1
  Constant/1 -> CompareToConstant/1
  CompareToConstant/1 -> Logical Operator/2
  Logical Operator/1 -> Offset/Enable
  state_Engine/1 -> Offset/1(state_Engine)
  state_Console/1 -> Offset/2(state_Console)
  position_OffsetPot/1 -> Offset/3(position_OffsetPot)
  state_OffsetPot/1 -> Offset/4(state_OffsetPot)
  calc_Vbattery/1 -> Offset/5(calc_Vbattery)
  state_Vbattery/1 -> Offset/6(state_Vbattery)
  afbk_A2D_OffsetBase/1 -> Offset/7(afbk_A2D_OffsetBase)
  ifbk_A2D_OffsetBase/1 -> Offset/8(ifbk_A2D_OffsetBase)
  afbk_A2D_OffsetRod/1 -> Offset/9(afbk_A2D_OffsetRod)
  ifbk_A2D_OffsetRod/1 -> Offset/10(ifbk_A2D_OffsetRod)
  Offset/1(hs_OffsetBase) -> hs_OffsetBase/1
  Offset/2(ls_OffsetBase) -> ls_OffsetBase/1
  Offset/3(errorCC_OffsetBase) -> errorCC_OffsetBase/1
  Offset/4(calc_ifbk_OffsetBase) -> calc_ifbk_OffsetBase/1
  Offset/5(state_OffsetBase) -> state_OffsetBase/1
  Offset/6(hs_OffsetRod) -> hs_OffsetRod/1
  Offset/7(ls_OffsetRod) -> ls_OffsetRod/1
  Offset/8(errorCC_OffsetRod) -> errorCC_OffsetRod/1
  Offset/9(calc_ifbk_OffsetRod) -> calc_ifbk_OffsetRod/1
  Offset/10(state_OffsetRod) -> state_OffsetRod/1
  ain_PrecRef/1 -> Offset/11(ain_PrecRef)
  mode_LeftControl/1 -> Offset/12(mode_LeftControl)
  mode_RightControl/1 -> Offset/13(mode_RightControl)
  position_LeftPot/1 -> Offset/14(position_LeftPot)
  state_LeftPot/1 -> Offset/15(state_LeftPot)
  position_RightPot/1 -> Offset/16(position_RightPot)
  state_RightPot/1 -> Offset/17(state_RightPot)
  state_Diverter/1 -> Offset/18(state_Diverter)
  flow_Aux2Percent/1 -> Offset/19(flow_Aux2Percent)
  flow_OffsetPercent/1 -> Offset/20(flow_OffsetPercent)

== system MX_Gateway/MX_Gtwy_Control/Outputs/PB_Valve ==
  dfbk_PBValveOutput : Inport  OutDataTypeStr=boolean
  din_PBValveRelay : Inport  Port=2  OutDataTypeStr=boolean
  adin_TravelPressureSwitch : Inport  Port=3
  aux_Flow : Inport  Port=4  OutDataTypeStr=uint8
  state_VBattery : Inport  Port=5
  state_AuxFlow : Inport  Port=6  OutDataTypeStr=uint8
  Compare To Constant : simulink/Logic and Bit Operations/Compare To Constant  relop=>  const=512  OutDataTypeStr=boolean
  Compare To Constant1 : simulink/Logic and Bit Operations/Compare To Constant  relop=~=  const=NON_FUNCTIONAL  OutDataTypeStr=boolean  ZeroCross=off
  Compare To Constant2 : simulink/Logic and Bit Operations/Compare To Constant  relop=~=  const=OORL  OutDataTypeStr=boolean  ZeroCross=off
  Compare To Constant3 : simulink/Logic and Bit Operations/Compare To Constant  relop=~=  const=NON_FUNCTIONAL  OutDataTypeStr=boolean  ZeroCross=off
  Compare To Constant4 : simulink/Logic and Bit Operations/Compare To Constant  relop=~=  const=OORL  OutDataTypeStr=boolean  ZeroCross=off
  Light1FeedbackState : Constant  Value=CD_PBVALVE_FEEDBACK_FUNCTION  OutDataTypeStr=uint8
  Light1FeedbackState1 : Constant  Value=CD_PBVALVE_RELAY_FEEDBACK_FUNCTION  OutDataTypeStr=uint8
  Logical Operator1 : Logic  AllPortsSameDT=off  OutDataTypeStr=boolean
  Logical Operator2 : Logic  AllPortsSameDT=off  OutDataTypeStr=boolean
  Logical Operator3 : Logic  Operator=NOT  AllPortsSameDT=off  OutDataTypeStr=boolean
  PBValve : Stateflow chart  TreatAsAtomicUnit=on
  Switch : Switch  Criteria=u2 ~= 0
  Unit Delay1 : UnitDelay  InitialCondition=NORMAL  SampleTime=-1
      settings: AttributesFormatString=<initial=%<X0>>\n<tsample=%<SampleTime>>
  Unit Delay3 : UnitDelay  InitialCondition=NORMAL  SampleTime=-1
      settings: AttributesFormatString=<initial=%<X0>>\n<tsample=%<SampleTime>>
  dout_chk : MX_Library/dout_chk  ERR_ON_DB_CNT=CD_PBVALVE_DEBOUNCE_ERROR_ON  ERR_OFF_DB_CNT=CD_PBVALVE_DEBOUNCE_ERROR_OFF
  relay_oc : MX_Library/relay_oc  ERROR_ON_DB_CNT=CD_PBVALVE_DEBOUNCE_ERROR_ON  ERROR_OFF_DB_CNT=CD_PBVALVE_DEBOUNCE_ERROR_OFF  NORMAL_DB_CNT=CD_PBVALVE_DEBOUNCE_NORMAL
  dout_PBValve : Outport
  state_PBValveOutput : Outport  Port=2
  state_PBValveRelay : Outport  Port=3
  wires:
  PBValve/1(dout_PBValve) -> dout_chk/1, dout_PBValve/1, relay_oc/1
  Logical Operator2/1 -> dout_chk/Enable  "ErrorDetectionEnable"
  Light1FeedbackState/1 -> Compare To Constant1/1
  [state_VBattery] -> Compare To Constant2/1  "state_VBattery"
  Compare To Constant1/1 -> Logical Operator2/1
  Compare To Constant2/1 -> Logical Operator2/2
  aux_Flow/1 -> PBValve/1(aux_Flow)
  din_PBValveRelay/1 -> relay_oc/3
  Light1FeedbackState1/1 -> Compare To Constant3/1
  [state_VBattery] -> Compare To Constant4/1  "state_VBattery"
  Compare To Constant3/1 -> Logical Operator1/1
  Compare To Constant4/1 -> Logical Operator1/2
  Logical Operator1/1 -> relay_oc/Enable, Logical Operator3/1
  relay_oc/2 -> state_PBValveRelay/1, [state_PBValveRelay]
  Switch/1 -> state_PBValveOutput/1, [state_PBValveOutput]
  dout_chk/1 -> Switch/1
  relay_oc/1 -> Switch/3
  Logical Operator3/1 -> Switch/2
  dfbk_PBValveOutput/1 -> [dfbk_PBValveOutput]
  [dfbk_PBValveOutput] -> relay_oc/2
  [dfbk_PBValveOutput] -> dout_chk/2
  Unit Delay3/1 -> PBValve/3(state_PBValveOutput)
  [state_PBValveOutput] -> Unit Delay3/1
  [state_PBValveRelay] -> Unit Delay1/1
  Unit Delay1/1 -> PBValve/4(state_PBValveRelay)
  adin_TravelPressureSwitch/1 -> Compare To Constant/1
  Compare To Constant/1 -> PBValve/2(din_TravelPressureSwitch)
  state_VBattery/1 -> [state_VBattery]
  state_AuxFlow/1 -> PBValve/5(state_AuxFlow)

== system MX_Gateway/MX_Gtwy_Control/Outputs/Starter ==
  state_Engine : Inport
  state_VBattery : Inport  Port=2
  flt_EngineSpeed : Inport  Port=3  OutDataTypeStr=uint16
  dfbk_StarterOutput : Inport  Port=4
  din_StarterRelay : Inport  Port=5
  ecu_EOT : Inport  Port=6  OutDataTypeStr=uint16
  state_FuelPriOutput : Inport  Port=7  OutDataTypeStr=uint8
  error_FuelSec : Inport  Port=8  OutDataTypeStr=uint8
  state_FuelPriRelay : Inport  Port=9  OutDataTypeStr=uint8
  Compare To Constant : simulink/Logic and Bit Operations/Compare To Constant  relop=~=  const=NON_FUNCTIONAL  OutDataTypeStr=uint8  ZeroCross=off
  Compare To Constant1 : simulink/Logic and Bit Operations/Compare To Constant  relop=~=  const=OORL  OutDataTypeStr=uint8  ZeroCross=off
  FunctionState : Constant  Value=CD_STARTER_FEEDBACK_FUNCTION  OutDataTypeStr=uint8
  Logical Operator1 : Logic  AllPortsSameDT=off  OutDataTypeStr=boolean
  Starter : Stateflow chart  TreatAsAtomicUnit=on
  Starter_Disenage_RPM : Lookup  InputValues=MXGTW_STARTER_AUTOMATED_OIL_TEMP  Table=MXGTW_STARTER_AUTOMATED_RPM_DISENGAGE_LEVEL  LookUpMeth=Use Input Below  OutDataTypeStr=uint16  SaturateOnIntegerOverflow=on
      settings: AttributesFormatString=input = %<InputValues>\noutput = %<OutputValues>
  Starter_Engage_RPM : Lookup  InputValues=MXGTW_STARTER_AUTOMATED_OIL_TEMP  Table=MXGTW_STARTER_AUTOMATED_RPM_ENGAGE_LEVEL  LookUpMeth=Use Input Below  OutDataTypeStr=uint16  SaturateOnIntegerOverflow=on
      settings: AttributesFormatString=input = %<InputValues>\noutput = %<OutputValues>
  Starter_Max_Time : Lookup  InputValues=MXGTW_STARTER_AUTOMATED_OIL_TEMP  Table=MXGTW_STARTER_AUTOMATED_MAX_TIME  LookUpMeth=Use Input Below  OutDataTypeStr=uint16  SaturateOnIntegerOverflow=on
      settings: AttributesFormatString=input = %<InputValues>\noutput = %<OutputValues>
  Unit Delay : UnitDelay  InitialCondition=8  SampleTime=-1
  Unit Delay1 : UnitDelay  InitialCondition=8  SampleTime=-1
  relay_oc : MX_Library/relay_oc  ERROR_ON_DB_CNT=CD_STARTER_DEBOUNCE_ERROR_ON  ERROR_OFF_DB_CNT=CD_STARTER_DEBOUNCE_ERROR_OFF  NORMAL_DB_CNT=CD_STARTER_DEBOUNCE_NORMAL
  dout_Starter : Outport  OutDataTypeStr=boolean
  state_StarterOutput : Outport  Port=2  OutDataTypeStr=uint8
  state_StarterRelay : Outport  Port=3  OutDataTypeStr=uint8
  wires:
  flt_EngineSpeed/1 -> Starter/11(flt_EngineSpeed)  "flt_EngineSpeed"
  Starter_Max_Time/1 -> Starter/10(time_StarterAutomated)  "time_StarterAutomated"
  Starter_Disenage_RPM/1 -> Starter/9(level_StarterAutomatedDisengage)  "level_StarterAutomatedDisengage"
  Starter_Engage_RPM/1 -> Starter/8(level_StarterAutomatedEnagage)  "level_StarterAutomatedEngage"
  ecu_EOT/1 -> Starter_Engage_RPM/1, Starter_Max_Time/1, Starter_Disenage_RPM/1  "ecu_EOT"
  [state_VBattery] -> Starter/2(state_VBattery)  "state_VBattery"
  state_Engine/1 -> Starter/1(state_Engine)  "state_Engine"
  Starter/1(dout_Starter) -> relay_oc/1, dout_Starter/1  "dout_Starter"
  state_FuelPriOutput/1 -> Starter/6(state_FuelPriOutput)  "state_FuelPriOutput"
  error_FuelSec/1 -> Starter/3(error_FuelSec)  "error_FuelSec"
  dfbk_StarterOutput/1 -> relay_oc/2  "dfbk_StarterOutput"
  din_StarterRelay/1 -> relay_oc/3  "din_StarterRelay"
  relay_oc/1 -> state_StarterOutput/1, Unit Delay/1  "state_StarterOutput"
  relay_oc/2 -> Unit Delay1/1, state_StarterRelay/1  "state_StarterRelay"
  FunctionState/1 -> Compare To Constant/1
  [state_VBattery] -> Compare To Constant1/1  "state_VBattery"
  Compare To Constant/1 -> Logical Operator1/1  "state_Feedback"
  Compare To Constant1/1 -> Logical Operator1/2  "state_VBatteryNOTOORL"
  Logical Operator1/1 -> relay_oc/Enable  "EnableFeedback"
  state_VBattery/1 -> [state_VBattery]  "state_VBattery"
  state_FuelPriRelay/1 -> Starter/7(state_FuelPriRelay)  "state_FuelPriRelay"
  Unit Delay/1 -> Starter/4(state_StarterOutput)  "state_StarterOutput"
  Unit Delay1/1 -> Starter/5(state_StarterRelay)  "state_StarterRelay"

== system MX_Gateway/MX_Gtwy_Control/Outputs/Starter_old ==
  state_Engine : Inport
  state_VBattery : Inport  Port=2
  dfbk_StarterOutput : Inport  Port=3
  din_StarterRelay : Inport  Port=4
  state_FuelPriOutput : Inport  Port=5  OutDataTypeStr=uint8
  error_FuelSec : Inport  Port=6  OutDataTypeStr=uint8
  state_FuelPriRelay : Inport  Port=7  OutDataTypeStr=uint8
  Compare To Constant : simulink/Logic and Bit Operations/Compare To Constant  relop=~=  const=NON_FUNCTIONAL  OutDataTypeStr=boolean  ZeroCross=off
  Compare To Constant1 : simulink/Logic and Bit Operations/Compare To Constant  relop=~=  const=OORL  OutDataTypeStr=boolean  ZeroCross=off
  FunctionState : Constant  Value=CD_STARTER_FEEDBACK_FUNCTION  OutDataTypeStr=uint8
  Logical Operator1 : Logic  AllPortsSameDT=off  OutDataTypeStr=boolean
  Starter : Stateflow chart  TreatAsAtomicUnit=on
  Unit Delay : UnitDelay  InitialCondition=8  SampleTime=-1
      settings: AttributesFormatString=Initial Ouput = %<X0>
  Unit Delay1 : UnitDelay  InitialCondition=8  SampleTime=-1
      settings: AttributesFormatString=Initial Ouput = %<X0>
  relay_oc : MX_Library/relay_oc  ERROR_ON_DB_CNT=CD_STARTER_DEBOUNCE_ERROR_ON  ERROR_OFF_DB_CNT=CD_STARTER_DEBOUNCE_ERROR_OFF  NORMAL_DB_CNT=CD_STARTER_DEBOUNCE_NORMAL
  dout_Starter : Outport  OutDataTypeStr=boolean
  state_StarterOutput : Outport  Port=2  OutDataTypeStr=uint8
  state_StarterRelay : Outport  Port=3  OutDataTypeStr=uint8
  wires:
  Unit Delay1/1 -> Starter/5(state_StarterRelay)  "state_StarterRelay"
  Unit Delay/1 -> Starter/4(state_StarterOutput)  "state_StarterOutput"
  state_FuelPriRelay/1 -> Starter/7(state_FuelPriRelay)  "state_FuelPriRelay"
  state_VBattery/1 -> [state_VBattery]  "state_VBattery"
  Logical Operator1/1 -> relay_oc/Enable  "EnableFeedback"
  Compare To Constant1/1 -> Logical Operator1/2  "state_VBatteryNOTOORL"
  Compare To Constant/1 -> Logical Operator1/1  "state_Feedback"
  [state_VBattery] -> Compare To Constant1/1  "state_VBattery"
  FunctionState/1 -> Compare To Constant/1
  relay_oc/2 -> Unit Delay1/1, state_StarterRelay/1  "state_StarterRelay"
  relay_oc/1 -> Unit Delay/1, state_StarterOutput/1  "state_StarterOutput"
  din_StarterRelay/1 -> relay_oc/3  "din_StarterRelay"
  dfbk_StarterOutput/1 -> relay_oc/2  "dfbk_StarterOutput"
  error_FuelSec/1 -> Starter/3(error_FuelSec)  "error_FuelSec"
  state_FuelPriOutput/1 -> Starter/6(state_FuelPriOutput)  "state_FuelPriOutput"
  Starter/1(dout_Starter) -> dout_Starter/1, relay_oc/1  "dout_Starter"
  state_Engine/1 -> Starter/1(state_Engine)  "state_Engine"
  [state_VBattery] -> Starter/2(state_VBattery)  "state_VBattery"

== system MX_Gateway/MX_Gtwy_Control/Outputs/SwitchedPower ==
  dkbk_Output : Inport
  din_Relay : Inport  Port=2
  din_RemoteKey : Inport  Port=3
  state_System : Inport  Port=4
  state_VBattery : Inport  Port=5
  state_Key : Inport  Port=6
  Compare To Constant1 : simulink/Logic and Bit Operations/Compare To Constant  relop=~=  const=OORL  OutDataTypeStr=boolean  ZeroCross=off
  Compare To Constant2 : simulink/Logic and Bit Operations/Compare To Constant  relop=~=  const=NON_FUNCTIONAL  OutDataTypeStr=boolean  ZeroCross=off
  Compare To Constant3 : simulink/Logic and Bit Operations/Compare To Constant  relop=~=  const=SYS_POWER_DOWN  OutDataTypeStr=boolean  ZeroCross=off
  Constant : Constant  Value=CD_SWITCHED_POWER_RELAY_FEEDBACK_FUNCTION  OutDataTypeStr=uint8
  Logical Operator : Logic  Inputs=3  AllPortsSameDT=off  OutDataTypeStr=boolean
  Relay : Stateflow chart  TreatAsAtomicUnit=on
  relay_oc : MX_Library/relay_oc  ERROR_ON_DB_CNT=CD_SWITCHED_POWER_RELAY_DEBOUNCE_ERROR_ON  ERROR_OFF_DB_CNT=CD_SWITCHED_POWER_RELAY_DEBOUNCE_ERROR_OFF  NORMAL_DB_CNT=CD_SWITCHED_POWER_RELAY_DEBOUNCE_NORMAL
  dout_SwitchedPwr : Outport
  state_SwitchedPwrOutput : Outport  Port=2
  state_SwitchedPwrRelay : Outport  Port=3
  wires:
  state_Key/1 -> Relay/1(state_Key)  "state_Key"
  din_RemoteKey/1 -> Relay/2(din_RemoteKey)  "din_RemoteKey"
  Relay/1(dout_SwitchedPwr) -> dout_SwitchedPwr/1, relay_oc/1  "dout_SwitchedPwr"
  Constant/1 -> Compare To Constant2/1
  Compare To Constant2/1 -> Logical Operator/1
  Logical Operator/1 -> relay_oc/Enable  "enable_Error"
  Compare To Constant1/1 -> Logical Operator/2
  state_VBattery/1 -> Compare To Constant1/1  "state_Battery"
  relay_oc/1 -> state_SwitchedPwrOutput/1  "state_SwitchedPwrOutput"
  relay_oc/2 -> state_SwitchedPwrRelay/1  "state_SwitchedPwrRelay"
  dkbk_Output/1 -> relay_oc/2  "dfbk_Output"
  din_Relay/1 -> relay_oc/3  "dfbk_Relay"
  [state_System] -> Compare To Constant3/1  "state_System"
  Compare To Constant3/1 -> Logical Operator/3
  state_System/1 -> [state_System]  "state_System"
  [state_System] -> Relay/3(state_System)  "state_System"
